simulink model "adc_soc_software_sync_am263x"  (R2024b)
settings: Solver=FixedStepAuto  StartTime=0.0  StopTime=10.0  RelTol=1e-3  EnableMultiTasking=on
libraries: peripheral_library_blocks_am263x x15, HWI_lib x1
settings Model: Creator=a0499511  LastModifiedBy=a1255762
settings OptimizationCC: DefaultParameterBehavior=Inlined  EnhancedBackFolding=on  PassReuseOutputArgsAs=Individual arguments  ZeroExternalMemoryAtStartup=off  ZeroInternalMemoryAtStartup=off  MaxStackSize=64  OptimizeBlockOrder=speed  DifferentSizesBufferReuse=on  OptimizationCustomize=off  EfficientTunableParamExpr=on
settings DebuggingCC: ParameterTunabilityLossMsg=error
settings HardwareCC: ProdBitPerPointer=32  ProdBitPerSizeT=32  ProdBitPerPtrDiffT=32  ProdLargestAtomicInteger=Long  ProdLargestAtomicFloat=Double  ProdWordSize=32  ProdLongLongMode=on  ProdHWDeviceType=ARM Compatible->ARM Cortex
settings SFSimCC: MATLABDynamicMemAlloc=off
settings RTWCC: Description=Embedded Coder  SystemTargetFile=ert.tlc  HardwareBoard=AM263X LP  TemplateMakefile=ert_default_tmf  Toolchain=TI ARM Clang Compiler AM263X | gmake
settings CodeAppCC: ForceParamTrailComments=on  IgnoreCustomStorageClasses=off  MaxIdLength=256  OperatorAnnotations=on  SimulinkDataObjDesc=on  SFDataObjDesc=on  InsertBlockDesc=on
settings ERTTargetCC: SupportVariableSizeSignals=on  ExtModeTransport=3  ExtModeStaticAlloc=on  ExtModeMexFile=ext_xcp  ExtModeIntrfLevel=Level2 - Open  ERTCustomFileTemplate=AM263x_codertarget_file_process.tlc
settings ConfigComp: CovBoundaryAbsTol=1.0e-5

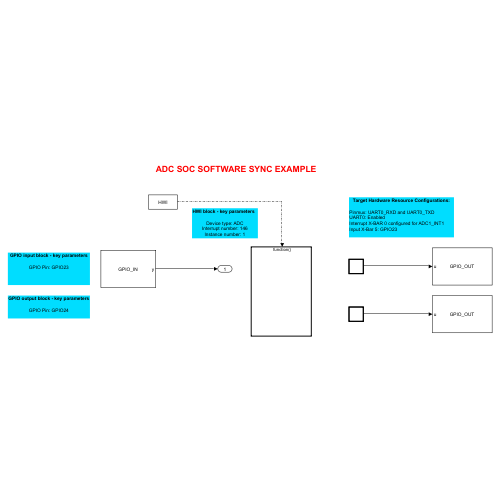
[thumbnail: Simulink's stored preview of the top-level diagram]
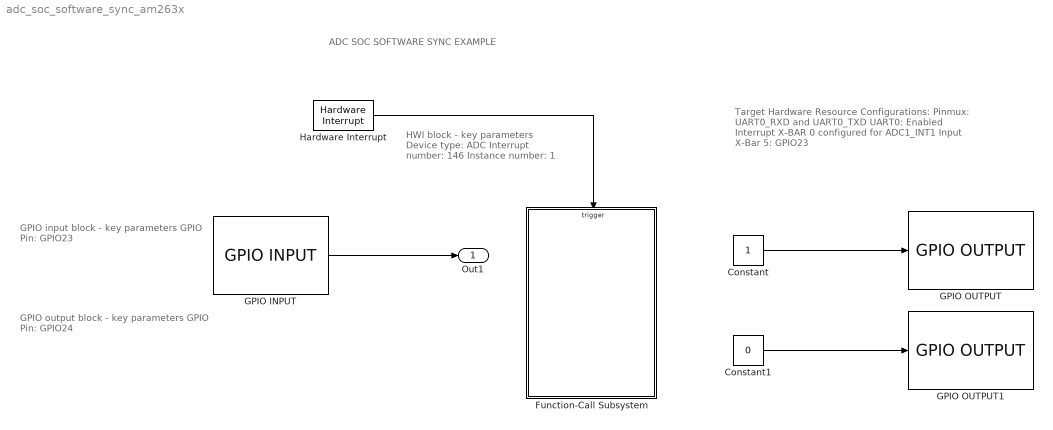
== system adc_soc_software_sync_am263x ==
  note: HWI block - key parameters
      |
      | Device type: ADC
      | Interrupt number: 146
      | Instance number: 1
  note: Target Hardware Resource Configurations:
      |
      | Pinmux: UART0_RXD and UART0_TXD
      | UART0: Enabled
      | Interrupt X-BAR 0 configured for ADC1_INT1
      | Input X-Bar 5: GPIO23
  note: ADC SOC SOFTWARE SYNC EXAMPLE
  note: GPIO input block - key parameters
      |
      | GPIO Pin: GPIO23
  note: GPIO output block - key parameters
      |
      | GPIO Pin: GPIO24
  Constant : Constant
  Constant1 : Constant  Value=0
  Function-Call Subsystem : Subsystem  TreatAsAtomicUnit=on
  GPIO INPUT : peripheral_library_blocks_am263x/GPIO INPUT  GPIO_Pin=GPIO23  Trigger_Type=None  GPIOEnableInterrupt=off  Output_DataType=Double  SampleTime=0.1
      settings: SourceProductName=Embedded Coder Support Package for AM263X - Peripheral Blocks
  GPIO OUTPUT : peripheral_library_blocks_am263x/GPIO OUTPUT  GPIO_Pin=GPIO24  Trigger_Type=None  GPIOEnableInterrupt=off
      settings: SourceProductName=Embedded Coder Support Package for AM263X - Peripheral Blocks
  GPIO OUTPUT1 : peripheral_library_blocks_am263x/GPIO OUTPUT  GPIO_Pin=GPIO24  Trigger_Type=None  GPIOEnableInterrupt=off
      settings: SourceProductName=Embedded Coder Support Package for AM263X - Peripheral Blocks
  Hardware Interrupt : HWI_lib/Hardware Interrupt  INTERRUPT_TYPE=ADC  ISR_NUMBER=146  INSTANCE_NUMBER=1  SHOW_INPUTPORT=off
      settings: SourceProductName=Embedded Coder Support Package for AM263x - HWI Block
  Out1 : Outport
  wires:
  Hardware Interrupt/1 -> Function-Call Subsystem/Trigger
  Constant/1 -> GPIO OUTPUT/1
  Constant1/1 -> GPIO OUTPUT1/1
  GPIO INPUT/1 -> Out1/1

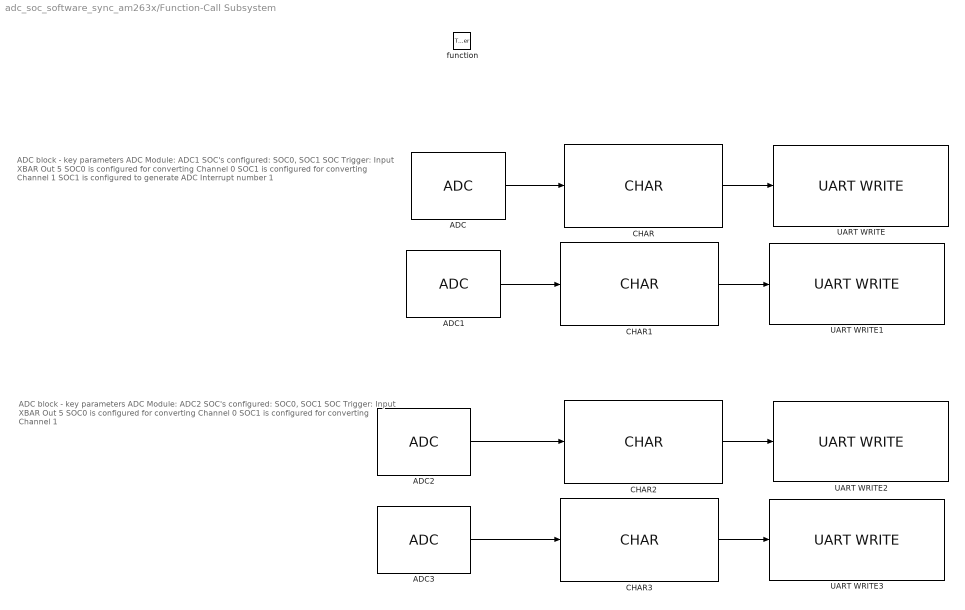
== system adc_soc_software_sync_am263x/Function-Call Subsystem ==
  note: ADC block - key parameters
      |
      | ADC Module: ADC2
      | SOC's configured: SOC0, SOC1
      | SOC Trigger: Input XBAR Out 5
      | SOC0 is configured for converting Channel 0
      | SOC1 is configured for converting Channel 1
  note: ADC block - key parameters
      |
      | ADC Module: ADC1
      | SOC's configured: SOC0, SOC1
      | SOC Trigger: Input XBAR Out 5
      | SOC0 is configured for converting Channel 0
      | SOC1 is configured for converting Channel 1
      | SOC1 is configured to generate ADC Interrupt number 1
  function : TriggerPort  TriggerType=function-call  VariantControl=(inherit)
  ADC : peripheral_library_blocks_am263x/ADC  ADCModule=ADC1  Prescaler=3.0  ResolutionMode=Twelve bit conversion  SOCPriority=All Round Robin  SignalMode=Sample on single pin with VREFLO  SOCTriggerNo=SOC0  SOCx_acquisition_Window=uint32(16)  SOCxTriggerSource=Input XBAR Out5  INTWillTriggerSOCx=No ADCINT Trigger  DataType=Unsigned INT16  ADCEnableConverter=on  PulseMode=Pulse generation at End of Acquisition Window  PostInterruptAtEOCTrigger=off  InterruptSelection=ADC Interrupt Number 1  InterruptSOCSource=SOC0  ADCINT1CONTINOUSMODE=off  ADCINT2CONTINOUSMODE=off  ADCINT3CONTINOUSMODE=off  ADCINT4CONTINOUSMODE=off  SampleTime=-1  Channels=Channel 0  Channels1=Channels 0 and 1  PPBBlock=PPB Block 1  PPBSocEocNO=SOC0  Enable_Event_ADC_Trip_High=off  Enable_Event_ADC_Trip_Low=off  Enable_Event_ADC_Zero_Crossing=off  Enable_Interrupt_Event_ADC_Trip_High=off  Enable_Interrupt_Event_ADC_Trip_Low=off  Enable_Interrupt_Event_ADC_Zero_Crossing=off  PPBEnableTwoComplement=off  PPBEnablePPBEventCBCClear=off  PPB_Calibration_Offset=0  PPB_Reference_Offset=0  PPB_High_Trip_Limit=0  PPB_Low_Trip_Limit=0  BurstModeTrigger=Software Source  BurstSize=1 SOC long  ADCEnableBurstMode=off
      settings: SourceProductName=Embedded Coder Support Package for AM263X - Peripheral Blocks
  ADC1 : peripheral_library_blocks_am263x/ADC  ADCModule=ADC1  Prescaler=3.0  ResolutionMode=Twelve bit conversion  SOCPriority=All Round Robin  SignalMode=Sample on single pin with VREFLO  SOCTriggerNo=SOC1  SOCx_acquisition_Window=uint32(16)  SOCxTriggerSource=Input XBAR Out5  INTWillTriggerSOCx=No ADCINT Trigger  DataType=Unsigned INT16  ADCEnableConverter=on  PulseMode=Pulse generation at End of Acquisition Window  PostInterruptAtEOCTrigger=on  InterruptSelection=ADC Interrupt Number 1  InterruptSOCSource=SOC1  ADCINT1CONTINOUSMODE=off  ADCINT2CONTINOUSMODE=off  ADCINT3CONTINOUSMODE=off  ADCINT4CONTINOUSMODE=off  SampleTime=-1  Channels=Channel 1  Channels1=Channels 0 and 1  PPBBlock=PPB Block 1  PPBSocEocNO=SOC0  Enable_Event_ADC_Trip_High=off  Enable_Event_ADC_Trip_Low=off  Enable_Event_ADC_Zero_Crossing=off  Enable_Interrupt_Event_ADC_Trip_High=off  Enable_Interrupt_Event_ADC_Trip_Low=off  Enable_Interrupt_Event_ADC_Zero_Crossing=off  PPBEnableTwoComplement=off  PPBEnablePPBEventCBCClear=off  PPB_Calibration_Offset=0  PPB_Reference_Offset=0  PPB_High_Trip_Limit=0  PPB_Low_Trip_Limit=0  BurstModeTrigger=Software Source  BurstSize=1 SOC long  ADCEnableBurstMode=off
      settings: SourceProductName=Embedded Coder Support Package for AM263X - Peripheral Blocks
  ADC2 : peripheral_library_blocks_am263x/ADC  ADCModule=ADC2  Prescaler=3.0  ResolutionMode=Twelve bit conversion  SOCPriority=All Round Robin  SignalMode=Sample on single pin with VREFLO  SOCTriggerNo=SOC0  SOCx_acquisition_Window=uint32(16)  SOCxTriggerSource=Input XBAR Out5  INTWillTriggerSOCx=No ADCINT Trigger  DataType=Unsigned INT16  ADCEnableConverter=on  PulseMode=Pulse generation at End of Acquisition Window  PostInterruptAtEOCTrigger=off  InterruptSelection=ADC Interrupt Number 1  InterruptSOCSource=SOC0  ADCINT1CONTINOUSMODE=off  ADCINT2CONTINOUSMODE=off  ADCINT3CONTINOUSMODE=off  ADCINT4CONTINOUSMODE=off  SampleTime=-1  Channels=Channel 0  Channels1=Channels 0 and 1  PPBBlock=PPB Block 1  PPBSocEocNO=SOC0  Enable_Event_ADC_Trip_High=off  Enable_Event_ADC_Trip_Low=off  Enable_Event_ADC_Zero_Crossing=off  Enable_Interrupt_Event_ADC_Trip_High=off  Enable_Interrupt_Event_ADC_Trip_Low=off  Enable_Interrupt_Event_ADC_Zero_Crossing=off  PPBEnableTwoComplement=off  PPBEnablePPBEventCBCClear=off  PPB_Calibration_Offset=0  PPB_Reference_Offset=0  PPB_High_Trip_Limit=0  PPB_Low_Trip_Limit=0  BurstModeTrigger=Software Source  BurstSize=1 SOC long  ADCEnableBurstMode=off
      settings: SourceProductName=Embedded Coder Support Package for AM263X - Peripheral Blocks
  ADC3 : peripheral_library_blocks_am263x/ADC  ADCModule=ADC2  Prescaler=3.0  ResolutionMode=Twelve bit conversion  SOCPriority=All Round Robin  SignalMode=Sample on single pin with VREFLO  SOCTriggerNo=SOC1  SOCx_acquisition_Window=uint32(16)  SOCxTriggerSource=Input XBAR Out5  INTWillTriggerSOCx=No ADCINT Trigger  DataType=Unsigned INT16  ADCEnableConverter=on  PulseMode=Pulse generation at End of Acquisition Window  PostInterruptAtEOCTrigger=off  InterruptSelection=ADC Interrupt Number 1  InterruptSOCSource=SOC0  ADCINT1CONTINOUSMODE=off  ADCINT2CONTINOUSMODE=off  ADCINT3CONTINOUSMODE=off  ADCINT4CONTINOUSMODE=off  SampleTime=-1  Channels=Channel 1  Channels1=Channels 0 and 1  PPBBlock=PPB Block 1  PPBSocEocNO=SOC0  Enable_Event_ADC_Trip_High=off  Enable_Event_ADC_Trip_Low=off  Enable_Event_ADC_Zero_Crossing=off  Enable_Interrupt_Event_ADC_Trip_High=off  Enable_Interrupt_Event_ADC_Trip_Low=off  Enable_Interrupt_Event_ADC_Zero_Crossing=off  PPBEnableTwoComplement=off  PPBEnablePPBEventCBCClear=off  PPB_Calibration_Offset=0  PPB_Reference_Offset=0  PPB_High_Trip_Limit=0  PPB_Low_Trip_Limit=0  BurstModeTrigger=Software Source  BurstSize=1 SOC long  ADCEnableBurstMode=off
      settings: SourceProductName=Embedded Coder Support Package for AM263X - Peripheral Blocks
  CHAR{,1..3} (4 alike) : peripheral_library_blocks_am263x/CHAR
      settings: SourceProductName=Embedded Coder Support Package for AM263X - Peripheral Blocks
  UART WRITE{,1..3} (4 alike) : peripheral_library_blocks_am263x/UART WRITE  UARTPort=UART0
      settings: SourceProductName=Embedded Coder Support Package for AM263X - Peripheral Blocks
  wires:
  ADC/1 -> CHAR/1
  CHAR/1 -> UART WRITE/1
  CHAR1/1 -> UART WRITE1/1
  ADC1/1 -> CHAR1/1
  CHAR3/1 -> UART WRITE3/1
  ADC3/1 -> CHAR3/1
  CHAR2/1 -> UART WRITE2/1
  ADC2/1 -> CHAR2/1
